simulink model "MX_Gateway"  (Simulink 9.0)
settings: Solver=FixedStepDiscrete  StartTime=0.0  StopTime=100  FixedStep=0.01  RelTol=1e-3
values from neighbouring files (symbols this model uses):
  ACTIVE = Bobcat.Parameter; CreateDefine( ACTIVE                         ,1                          ,'Define' ,'defines.h' )    [defines.m]
  ACTIVE_MODE_ONE = Bobcat.Parameter; CreateDefine( ACTIVE_MODE_ONE                ,19                         ,'Define' ,'defines.h' )    [defines.m]
  AUTHENTICATED = Bobcat.Parameter; CreateDefine( AUTHENTICATED                  ,1                          ,'Define' ,'defines.h' )    [defines.m]
  AUX_EMEA_CONTROLS = Bobcat.Parameter; CreateDefine( AUX_EMEA_CONTROLS  ,1   ,'Define' ,'defines.h' )    [enums.m]
  AUX_FLOW_HIGH = Bobcat.Parameter; CreateDefine( AUX_FLOW_HIGH           ,4  ,'Define' ,'defines.h' )    [enums.m]
  AUX_FLOW_INACTIVE = Bobcat.Parameter; CreateDefine( AUX_FLOW_INACTIVE       ,0  ,'Define' ,'defines.h' )    [enums.m]
  AUX_FLOW_LOW = Bobcat.Parameter; CreateDefine( AUX_FLOW_LOW            ,1  ,'Define' ,'defines.h' )    [enums.m]
  AUX_FLOW_MEDIUM = Bobcat.Parameter; CreateDefine( AUX_FLOW_MEDIUM         ,2  ,'Define' ,'defines.h' )    [enums.m]
  AUX_FLOW_STANDARD = Bobcat.Parameter; CreateDefine( AUX_FLOW_STANDARD       ,3  ,'Define' ,'defines.h' )    [enums.m]
  AUX_NON_FUNCTIONAL = Bobcat.Parameter; CreateDefine( AUX_NON_FUNCTIONAL ,255 ,'Define' ,'defines.h' )    [enums.m]
  BASE_ACTIVE = Bobcat.Parameter; CreateDefine( BASE_ACTIVE                    ,253                        ,'Define' ,'defines.h' )    [defines.m]
  BASE_DETENT = Bobcat.Parameter; CreateDefine( BASE_DETENT                    ,1                          ,'Define' ,'defines.h' )    [defines.m]
  BEEP_NONE = Bobcat.Parameter; CreateDefine( BEEP_NONE                      ,255                        ,'Define' ,'defines.h' )    [defines.m]
  CD_AIR_FILTER_DEBOUNCE_NORMAL = Bobcat.Parameter; CreateAliasCD( CD_AIR_FILTER_DEBOUNCE_NORMAL                        ,uint16(500)                ,'ConstantDataPointer' ,'CONSTANT_DATA' ,'AIR_FILTER_DEBOUNCE_NORMAL')    [cdata.m]    (also in hc12_cdata.m; m4_cdata.m)
  CD_AIR_FILTER_DEBOUNCE_NOT_CONNECTED = Bobcat.Parameter; CreateAliasCD( CD_AIR_FILTER_DEBOUNCE_NOT_CONNECTED                 ,uint16(3000)               ,'ConstantDataPointer' ,'CONSTANT_DATA' ,'AIR_FILTER_DEBOUNCE_NOT_CONNECTED')    [cdata.m]    (also in hc12_cdata.m; m4_cdata.m)
  CD_AIR_FILTER_DEBOUNCE_PLUGGED = Bobcat.Parameter; CreateAliasCD( CD_AIR_FILTER_DEBOUNCE_PLUGGED                       ,uint16(3000)               ,'ConstantDataPointer' ,'CONSTANT_DATA' ,'AIR_FILTER_DEBOUNCE_PLUGGED')    [cdata.m]    (also in hc12_cdata.m; m4_cdata.m)
  CD_AIR_FILTER_FUNCTION = Bobcat.Parameter; CreateAliasCD( CD_AIR_FILTER_FUNCTION                               ,uint8(255)                 ,'ConstantDataPointer' ,'CONSTANT_DATA' ,'AIR_FILTER_FUNCTION')    [cdata.m]    (also in hc12_cdata.m; m4_cdata.m)
  CD_AIR_FILTER_PLUGGED_WEIGHT = Bobcat.Parameter; CreateAliasCD( CD_AIR_FILTER_PLUGGED_WEIGHT                         ,uint8(2)                   ,'ConstantDataPointer' ,'CONSTANT_DATA' ,'AIR_FILTER_PLUGGED_WEIGHT')    [cdata.m]    (also in hc12_cdata.m; m4_cdata.m)
  CD_AIR_FILTER_UNPLUGGED_WEIGHT = Bobcat.Parameter; CreateAliasCD( CD_AIR_FILTER_UNPLUGGED_WEIGHT                       ,uint8(1)                   ,'ConstantDataPointer' ,'CONSTANT_DATA' ,'AIR_FILTER_UNPLUGGED_WEIGHT')    [cdata.m]    (also in hc12_cdata.m; m4_cdata.m)
  CD_AUTOIDLE_ARMBAR_DELAY = Bobcat.Parameter; CreateAliasCD( CD_AUTOIDLE_ARMBAR_DELAY                             ,uint8(2)                   ,'ConstantDataPointer' ,'CONSTANT_DATA' ,'AUTOIDLE_ARMBAR_DELAY')    [cdata.m]    (also in hc12_cdata.m; m4_cdata.m)
  CD_AUTOIDLE_FUNCTION = Bobcat.Parameter; CreateAliasCD( CD_AUTOIDLE_FUNCTION                                 ,uint8(255)                 ,'ConstantDataPointer' ,'CONSTANT_DATA' ,'AUTOIDLE_FUNCTION')    [cdata.m]    (also in hc12_cdata.m; m4_cdata.m)
  CD_AUTOIDLE_IDLE_DELAY = Bobcat.Parameter; CreateAliasCD( CD_AUTOIDLE_IDLE_DELAY                               ,uint8(40)                  ,'ConstantDataPointer' ,'CONSTANT_DATA' ,'AUTOIDLE_IDLE_DELAY')    [cdata.m]    (also in hc12_cdata.m; m4_cdata.m)
  CD_AUTOIDLE_LOW_IDLE_START = Bobcat.Parameter; CreateAliasCD( CD_AUTOIDLE_LOW_IDLE_START                           ,uint8(255)                 ,'ConstantDataPointer' ,'CONSTANT_DATA' ,'AUTOIDLE_LOW_IDLE_START')    [cdata.m]    (also in hc12_cdata.m; m4_cdata.m)
  CD_AUTOIDLE_PRESSURE_OFF = Bobcat.Parameter; CreateAliasCD( CD_AUTOIDLE_PRESSURE_OFF                             ,uint16(300)                ,'ConstantDataPointer' ,'CONSTANT_DATA' ,'AUTOIDLE_PRESSURE_OFF')    [cdata.m]    (also in hc12_cdata.m; m4_cdata.m)
  CD_AUTOIDLE_PRESSURE_ON = Bobcat.Parameter; CreateAliasCD( CD_AUTOIDLE_PRESSURE_ON                              ,uint16(270)                ,'ConstantDataPointer' ,'CONSTANT_DATA' ,'AUTOIDLE_PRESSURE_ON')    [cdata.m]    (also in hc12_cdata.m; m4_cdata.m)
  CD_AUTOSHIFT_FUNCTION = Bobcat.Parameter; CreateAliasCD( CD_AUTOSHIFT_FUNCTION                                ,uint8(255)                 ,'ConstantDataPointer' ,'CONSTANT_DATA' ,'AUTOSHIFT_FUNCTION')    [cdata.m]    (also in hc12_cdata.m; m4_cdata.m)
  CD_AUTOSHIFT_PHIGH1 = Bobcat.Parameter; CreateAliasCD( CD_AUTOSHIFT_PHIGH1                                  ,uint16(205)                ,'ConstantDataPointer' ,'CONSTANT_DATA' ,'AUTOSHIFT_PHIGH1')    [cdata.m]    (also in hc12_cdata.m; m4_cdata.m)
  CD_AUTOSHIFT_PHIGH2 = Bobcat.Parameter; CreateAliasCD( CD_AUTOSHIFT_PHIGH2                                  ,uint16(193)                ,'ConstantDataPointer' ,'CONSTANT_DATA' ,'AUTOSHIFT_PHIGH2')    [cdata.m]    (also in hc12_cdata.m; m4_cdata.m)
  CD_AUTOSHIFT_PHIGH_TEMP1 = Bobcat.Parameter; CreateAliasCD( CD_AUTOSHIFT_PHIGH_TEMP1                             ,int16(70)                  ,'ConstantDataPointer' ,'CONSTANT_DATA' ,'AUTOSHIFT_PHIGH_TEMP1')    [cdata.m]    (also in hc12_cdata.m; m4_cdata.m)
  CD_AUTOSHIFT_PHIGH_TEMP2 = Bobcat.Parameter; CreateAliasCD( CD_AUTOSHIFT_PHIGH_TEMP2                             ,int16(160)                 ,'ConstantDataPointer' ,'CONSTANT_DATA' ,'AUTOSHIFT_PHIGH_TEMP2')    [cdata.m]    (also in hc12_cdata.m; m4_cdata.m)
  CD_AUTOSHIFT_PLOW1 = Bobcat.Parameter; CreateAliasCD( CD_AUTOSHIFT_PLOW1                                   ,uint16(130)                ,'ConstantDataPointer' ,'CONSTANT_DATA' ,'AUTOSHIFT_PLOW1')    [cdata.m]    (also in hc12_cdata.m; m4_cdata.m)
  CD_AUTOSHIFT_PLOW2 = Bobcat.Parameter; CreateAliasCD( CD_AUTOSHIFT_PLOW2                                   ,uint16(100)                ,'ConstantDataPointer' ,'CONSTANT_DATA' ,'AUTOSHIFT_PLOW2')    [cdata.m]    (also in hc12_cdata.m; m4_cdata.m)
  CD_AUTOSHIFT_PLOW_TEMP1 = Bobcat.Parameter; CreateAliasCD( CD_AUTOSHIFT_PLOW_TEMP1                              ,int16(20)                  ,'ConstantDataPointer' ,'CONSTANT_DATA' ,'AUTOSHIFT_PLOW_TEMP1')    [cdata.m]    (also in hc12_cdata.m; m4_cdata.m)
  CD_AUTOSHIFT_PLOW_TEMP2 = Bobcat.Parameter; CreateAliasCD( CD_AUTOSHIFT_PLOW_TEMP2                              ,int16(80)                  ,'ConstantDataPointer' ,'CONSTANT_DATA' ,'AUTOSHIFT_PLOW_TEMP2')    [cdata.m]    (also in hc12_cdata.m; m4_cdata.m)
  CD_AUTOSHIFT_TRAVEL_DELAY = Bobcat.Parameter; CreateAliasCD( CD_AUTOSHIFT_TRAVEL_DELAY                            ,uint8(32)                  ,'ConstantDataPointer' ,'CONSTANT_DATA' ,'AUTOSHIFT_TRAVEL_DELAY')    [cdata.m]    (also in hc12_cdata.m; m4_cdata.m)
  CD_AUX2_CRACKPOINT_CURRENT = Bobcat.Parameter; CreateAliasCD( CD_AUX2_CRACKPOINT_CURRENT                           ,fi(0,0,16,12,'hex','0c28') ,'ConstantDataPointer' ,'CONSTANT_DATA' ,'AUX2_CRACKPOINT_CURRENT')    [cdata.m]    (also in hc12_cdata.m; m4_cdata.m)
  CD_AUX2_FLOW_LOW_FUNCTION = Bobcat.Parameter; CreateAliasCD( CD_AUX2_FLOW_LOW_FUNCTION                            ,uint8(255)                 ,'ConstantDataPointer' ,'CONSTANT_DATA' ,'AUX2_FLOW_LOW_FUNCTION')    [cdata.m]    (also in hc12_cdata.m; m4_cdata.m)
  CD_AUX2_FLOW_MED_FUNCTION = Bobcat.Parameter; CreateAliasCD( CD_AUX2_FLOW_MED_FUNCTION                            ,uint8(255)                 ,'ConstantDataPointer' ,'CONSTANT_DATA' ,'AUX2_FLOW_MED_FUNCTION')    [cdata.m]    (also in hc12_cdata.m; m4_cdata.m)
  CD_AUX2_HI_FLOW_PERCENT = Bobcat.Parameter; CreateAliasCD( CD_AUX2_HI_FLOW_PERCENT                              ,fi(0,0,16,16,'hex','ffff') ,'ConstantDataPointer' ,'CONSTANT_DATA' ,'AUX2_HI_FLOW_PERCENT')    [cdata.m]    (also in hc12_cdata.m; m4_cdata.m)
  CD_AUX2_LOW_FLOW_PERCENT = Bobcat.Parameter; CreateAliasCD( CD_AUX2_LOW_FLOW_PERCENT                             ,fi(0,0,16,16,'hex','7333') ,'ConstantDataPointer' ,'CONSTANT_DATA' ,'AUX2_LOW_FLOW_PERCENT')    [cdata.m]    (also in hc12_cdata.m; m4_cdata.m)
  CD_AUX2_MAX_FLOW_CURRENT = Bobcat.Parameter; CreateAliasCD( CD_AUX2_MAX_FLOW_CURRENT                             ,fi(0,0,16,12,'hex','14cc') ,'ConstantDataPointer' ,'CONSTANT_DATA' ,'AUX2_MAX_FLOW_CURRENT')    [cdata.m]    (also in hc12_cdata.m; m4_cdata.m)
  CD_AUX2_MED_FLOW_PERCENT = Bobcat.Parameter; CreateAliasCD( CD_AUX2_MED_FLOW_PERCENT                             ,fi(0,0,16,16,'hex','9999') ,'ConstantDataPointer' ,'CONSTANT_DATA' ,'AUX2_MED_FLOW_PERCENT')    [cdata.m]    (also in hc12_cdata.m; m4_cdata.m)
  CD_AUX_CRACKPOINT_CURRENT = Bobcat.Parameter; CreateAliasCD( CD_AUX_CRACKPOINT_CURRENT                            ,fi(0,0,16,12,'hex','0000') ,'ConstantDataPointer' ,'CONSTANT_DATA' ,'AUX_CRACKPOINT_CURRENT')    [cdata.m]    (also in hc12_cdata.m; m4_cdata.m)
  CD_AUX_DETENT_FLASH_TIME = Bobcat.Parameter; CreateAliasCD( CD_AUX_DETENT_FLASH_TIME                             ,uint16(72)                 ,'ConstantDataPointer' ,'CONSTANT_DATA' ,'AUX_DETENT_FLASH_TIME')    [cdata.m]    (also in hc12_cdata.m; m4_cdata.m)
  CD_AUX_DETENT_HOLD_TIME = Bobcat.Parameter; CreateAliasCD( CD_AUX_DETENT_HOLD_TIME                              ,uint16(100)                ,'ConstantDataPointer' ,'CONSTANT_DATA' ,'AUX_DETENT_HOLD_TIME')    [cdata.m]    (also in hc12_cdata.m; m4_cdata.m)
  CD_AUX_FULL_ON_AUX1 = Bobcat.Parameter; CreateAliasCD( CD_AUX_FULL_ON_AUX1                                  ,fi(0,0,16,12,'hex','10cd') ,'ConstantDataPointer' ,'CONSTANT_DATA' ,'AUX_FULL_ON_AUX1')    [cdata.m]    (also in hc12_cdata.m; m4_cdata.m)
  CD_AUX_FULL_ON_AUX2 = Bobcat.Parameter; CreateAliasCD( CD_AUX_FULL_ON_AUX2                                  ,fi(0,0,16,12,'hex','139d') ,'ConstantDataPointer' ,'CONSTANT_DATA' ,'AUX_FULL_ON_AUX2')    [cdata.m]    (also in hc12_cdata.m; m4_cdata.m)
  CD_AUX_FULL_ON_AUX3 = Bobcat.Parameter; CreateAliasCD( CD_AUX_FULL_ON_AUX3                                  ,fi(0,0,16,12,'hex','17d7') ,'ConstantDataPointer' ,'CONSTANT_DATA' ,'AUX_FULL_ON_AUX3')    [cdata.m]    (also in hc12_cdata.m; m4_cdata.m)
  CD_AUX_FUNCTION = Bobcat.Parameter; CreateAliasCD( CD_AUX_FUNCTION                                      ,uint8(0)                   ,'ConstantDataPointer' ,'CONSTANT_DATA' ,'AUX_FUNCTION')    [cdata.m]    (also in hc12_cdata.m; m4_cdata.m)
  CD_AUX_RATE_LIMIT_CRACKPOINT = Bobcat.Parameter; CreateAliasCD( CD_AUX_RATE_LIMIT_CRACKPOINT                         ,fi(0,0,16,12,'hex','0800') ,'ConstantDataPointer' ,'CONSTANT_DATA' ,'AUX_RATE_LIMIT_CRACKPOINT')    [cdata.m]    (also in hc12_cdata.m; m4_cdata.m)
  CD_AUX_RATE_LIMIT_CRACKPOINT_RATE = Bobcat.Parameter; CreateAliasCD( CD_AUX_RATE_LIMIT_CRACKPOINT_RATE                    ,fi(0,1,32,12,'int',102400) ,'ConstantDataPointer' ,'CONSTANT_DATA' ,'AUX_RATE_LIMIT_CRACKPOINT_RATE')    [cdata.m]    (also in hc12_cdata.m; m4_cdata.m)
  CD_AUX_RATE_LIMIT_FUNCTION = Bobcat.Parameter; CreateAliasCD( CD_AUX_RATE_LIMIT_FUNCTION                           ,uint8(255)                 ,'ConstantDataPointer' ,'CONSTANT_DATA' ,'AUX_RATE_LIMIT_FUNCTION')    [cdata.m]    (also in hc12_cdata.m; m4_cdata.m)
  CD_AUX_RATE_LIMIT_MAX_ENG_SPD = Bobcat.Parameter; CreateAliasCD( CD_AUX_RATE_LIMIT_MAX_ENG_SPD                        ,uint16(1600)               ,'ConstantDataPointer' ,'CONSTANT_DATA' ,'AUX_RATE_LIMIT_MAX_ENG_SPD')    [cdata.m]    (also in hc12_cdata.m; m4_cdata.m)
  CD_AUX_RATE_LIMIT_MIN_ENG_SPD = Bobcat.Parameter; CreateAliasCD( CD_AUX_RATE_LIMIT_MIN_ENG_SPD                        ,uint16(100)                ,'ConstantDataPointer' ,'CONSTANT_DATA' ,'AUX_RATE_LIMIT_MIN_ENG_SPD')    [cdata.m]    (also in hc12_cdata.m; m4_cdata.m)
  CD_AUX_RATE_LIMIT_NORMAL_RATE = Bobcat.Parameter; CreateAliasCD( CD_AUX_RATE_LIMIT_NORMAL_RATE                        ,fi(0,1,32,12,'int',2048)   ,'ConstantDataPointer' ,'CONSTANT_DATA' ,'AUX_RATE_LIMIT_NORMAL_RATE')    [cdata.m]    (also in hc12_cdata.m; m4_cdata.m)
  CD_AUXPOT_CAL_DEADBAND_HIGH = Bobcat.Parameter; CreateAliasCD( CD_AUXPOT_CAL_DEADBAND_HIGH                          ,uint16(574)                ,'ConstantDataPointer' ,'CONSTANT_DATA' ,'AUXPOT_CAL_DEADBAND_HIGH')    [cdata.m]    (also in hc12_cdata.m; m4_cdata.m)
  CD_AUXPOT_CAL_DEADBAND_LOW = Bobcat.Parameter; CreateAliasCD( CD_AUXPOT_CAL_DEADBAND_LOW                           ,uint16(424)                ,'ConstantDataPointer' ,'CONSTANT_DATA' ,'AUXPOT_CAL_DEADBAND_LOW')    [cdata.m]    (also in hc12_cdata.m; m4_cdata.m)
  CD_AUXPOT_CENTER_RATIO = Bobcat.Parameter; CreateAliasCD( CD_AUXPOT_CENTER_RATIO                               ,uint16(500)                ,'ConstantDataPointer' ,'CONSTANT_DATA' ,'AUXPOT_CENTER_RATIO')    [cdata.m]    (also in hc12_cdata.m; m4_cdata.m)
  CD_AUXPOT_DEADBAND = Bobcat.Parameter; CreateAliasCD( CD_AUXPOT_DEADBAND                                   ,uint8(38)                  ,'ConstantDataPointer' ,'CONSTANT_DATA' ,'AUXPOT_DEADBAND')    [cdata.m]    (also in hc12_cdata.m; m4_cdata.m)
  CD_AUXPOT_FUNCTION = Bobcat.Parameter; CreateAliasCD( CD_AUXPOT_FUNCTION                                   ,uint8(0)                   ,'ConstantDataPointer' ,'CONSTANT_DATA' ,'AUXPOT_FUNCTION')    [cdata.m]    (also in hc12_cdata.m; m4_cdata.m)
  CD_AUXPOT_RATIO_GAIN = Bobcat.Parameter; CreateAliasCD( CD_AUXPOT_RATIO_GAIN                                 ,uint16(1000)               ,'ConstantDataPointer' ,'CONSTANT_DATA' ,'AUXPOT_RATIO_GAIN')    [cdata.m]    (also in hc12_cdata.m; m4_cdata.m)
  CD_CAN_THROTTLE_FUNCTION = Bobcat.Parameter; CreateAliasCD( CD_CAN_THROTTLE_FUNCTION                             ,uint8(255)                 ,'ConstantDataPointer' ,'CONSTANT_DATA' ,'CAN_THROTTLE_FUNCTION')    [cdata.m]    (also in hc12_cdata.m; m4_cdata.m)
  CD_CAN_THROTTLE_REVERSE = Bobcat.Parameter; CreateAliasCD( CD_CAN_THROTTLE_REVERSE                              ,uint8(255)                 ,'ConstantDataPointer' ,'CONSTANT_DATA' ,'CAN_THROTTLE_REVERSE')    [cdata.m]    (also in hc12_cdata.m; m4_cdata.m)
  CD_CONSOLE_DEBOUNCE_ABSENT = Bobcat.Parameter; CreateAliasCD( CD_CONSOLE_DEBOUNCE_ABSENT                           ,uint16(12)                 ,'ConstantDataPointer' ,'CONSTANT_DATA' ,'CONSOLE_DEBOUNCE_ABSENT')    [cdata.m]    (also in hc12_cdata.m; m4_cdata.m)
  CD_CONSOLE_DEBOUNCE_OORH = Bobcat.Parameter; CreateAliasCD( CD_CONSOLE_DEBOUNCE_OORH                             ,uint16(12)                 ,'ConstantDataPointer' ,'CONSTANT_DATA' ,'CONSOLE_DEBOUNCE_OORH')    [cdata.m]    (also in hc12_cdata.m; m4_cdata.m)
  CD_CONSOLE_DEBOUNCE_OORL = Bobcat.Parameter; CreateAliasCD( CD_CONSOLE_DEBOUNCE_OORL                             ,uint16(12)                 ,'ConstantDataPointer' ,'CONSTANT_DATA' ,'CONSOLE_DEBOUNCE_OORL')    [cdata.m]    (also in hc12_cdata.m; m4_cdata.m)
  CD_CONSOLE_DEBOUNCE_PRESENT = Bobcat.Parameter; CreateAliasCD( CD_CONSOLE_DEBOUNCE_PRESENT                          ,uint16(12)                 ,'ConstantDataPointer' ,'CONSTANT_DATA' ,'CONSOLE_DEBOUNCE_PRESENT')    [cdata.m]    (also in hc12_cdata.m; m4_cdata.m)
  CD_CONSOLE_FUNCTION = Bobcat.Parameter; CreateAliasCD( CD_CONSOLE_FUNCTION                                  ,uint8(0)                   ,'ConstantDataPointer' ,'CONSTANT_DATA' ,'CONSOLE_FUNCTION')    [cdata.m]    (also in hc12_cdata.m; m4_cdata.m)
  CD_CONSOLE_OORH = Bobcat.Parameter; CreateAliasCD( CD_CONSOLE_OORH                                      ,uint16(740)                ,'ConstantDataPointer' ,'CONSTANT_DATA' ,'CONSOLE_OORH')    [cdata.m]    (also in hc12_cdata.m; m4_cdata.m)
  CD_CONSOLE_OORL = Bobcat.Parameter; CreateAliasCD( CD_CONSOLE_OORL                                      ,uint16(10)                 ,'ConstantDataPointer' ,'CONSTANT_DATA' ,'CONSOLE_OORL')    [cdata.m]    (also in hc12_cdata.m; m4_cdata.m)
  CD_CONSOLE_PRESENT_HIGH = Bobcat.Parameter; CreateAliasCD( CD_CONSOLE_PRESENT_HIGH                              ,uint16(515)                ,'ConstantDataPointer' ,'CONSTANT_DATA' ,'CONSOLE_PRESENT_HIGH')    [cdata.m]    (also in hc12_cdata.m; m4_cdata.m)
  CD_CONSOLE_PRESENT_LOW = Bobcat.Parameter; CreateAliasCD( CD_CONSOLE_PRESENT_LOW                               ,uint16(375)                ,'ConstantDataPointer' ,'CONSTANT_DATA' ,'CONSOLE_PRESENT_LOW')    [cdata.m]    (also in hc12_cdata.m; m4_cdata.m)
  CD_DELUXE_GEN5_5_FUNCTION = Bobcat.Parameter; CreateAliasCD( CD_DELUXE_GEN5_5_FUNCTION                            ,uint8(255)                 ,'ConstantDataPointer' ,'CONSTANT_DATA' ,'DELUXE_GEN5_5_FUNCTION')    [cdata.m]    (also in hc12_cdata.m; m4_cdata.m)
  CD_DELUXE_GEN5_FUNCTION = Bobcat.Parameter; CreateAliasCD( CD_DELUXE_GEN5_FUNCTION                              ,uint8(255)                 ,'ConstantDataPointer' ,'CONSTANT_DATA' ,'DELUXE_GEN5_FUNCTION')    [cdata.m]    (also in hc12_cdata.m; m4_cdata.m)
  CD_DERATE_ENGINE_SPEED_DEBOUNCE_HIGH = Bobcat.Parameter; CreateAliasCD( CD_DERATE_ENGINE_SPEED_DEBOUNCE_HIGH                 ,uint16(200)                ,'ConstantDataPointer' ,'CONSTANT_DATA' ,'DERATE_ENGINE_SPEED_DEBOUNCE_HIGH')    [cdata.m]    (also in hc12_cdata.m; m4_cdata.m)
  CD_DERATE_ENGINE_SPEED_DEBOUNCE_SHUTDOWN = Bobcat.Parameter; CreateAliasCD( CD_DERATE_ENGINE_SPEED_DEBOUNCE_SHUTDOWN             ,uint16(1000)               ,'ConstantDataPointer' ,'CONSTANT_DATA' ,'DERATE_ENGINE_SPEED_DEBOUNCE_SHUTDOWN')    [cdata.m]    (also in hc12_cdata.m; m4_cdata.m)
  CD_DERATE_ENGINE_SPEED_HIGH_OFFSET = Bobcat.Parameter; CreateAliasCD( CD_DERATE_ENGINE_SPEED_HIGH_OFFSET                   ,uint16(200)                ,'ConstantDataPointer' ,'CONSTANT_DATA' ,'DERATE_ENGINE_SPEED_HIGH_OFFSET')    [cdata.m]    (also in hc12_cdata.m; m4_cdata.m)
  CD_DERATE_METHOD = Bobcat.Parameter; CreateAliasCD( CD_DERATE_METHOD                                     ,uint8(0)                   ,'ConstantDataPointer' ,'CONSTANT_DATA' ,'DERATE_METHOD')    [cdata.m]    (also in hc12_cdata.m; m4_cdata.m)
  CD_DUMP_VALVE_FUNCTION = Bobcat.Parameter; CreateAliasCD( CD_DUMP_VALVE_FUNCTION                               ,uint8(255)                 ,'ConstantDataPointer' ,'CONSTANT_DATA' ,'DUMP_VALVE_FUNCTION')    [cdata.m]    (also in hc12_cdata.m; m4_cdata.m)
  CD_DUMP_VALVE_ON_CURRENT = Bobcat.Parameter; CreateAliasCD( CD_DUMP_VALVE_ON_CURRENT                             ,fi(0,0,16,12,'hex','ffff') ,'ConstantDataPointer' ,'CONSTANT_DATA' ,'DUMP_VALVE_ON_CURRENT')    [cdata.m]    (also in hc12_cdata.m; m4_cdata.m)
  CD_ECO_MODE_THROTTLE_REDUCTION = Bobcat.Parameter; CreateAliasCD( CD_ECO_MODE_THROTTLE_REDUCTION                       ,uint16(850)                ,'ConstantDataPointer' ,'CONSTANT_DATA' ,'ECO_MODE_THROTTLE_REDUCTION')    [cdata.m]    (also in hc12_cdata.m; m4_cdata.m)
  CD_ECT_FILTER_GAIN = Bobcat.Parameter; CreateAliasCD( CD_ECT_FILTER_GAIN                                   ,uint8(6)                   ,'ConstantDataPointer' ,'CONSTANT_DATA' ,'ECT_FILTER_GAIN')    [cdata.m]    (also in hc12_cdata.m; m4_cdata.m)
  CD_ECU_FUNCTION = Bobcat.Parameter; CreateAliasCD( CD_ECU_FUNCTION                                      ,uint8(255)                 ,'ConstantDataPointer' ,'CONSTANT_DATA' ,'ECU_FUNCTION')    [cdata.m]    (also in hc12_cdata.m; m4_cdata.m)
  CD_ECU_HOLD_DEBOUNCE_ERROR_ON = Bobcat.Parameter; CreateAliasCD( CD_ECU_HOLD_DEBOUNCE_ERROR_ON                        ,uint16(20)                 ,'ConstantDataPointer' ,'CONSTANT_DATA' ,'ECU_HOLD_DEBOUNCE_ERROR_ON')    [cdata.m]    (also in hc12_cdata.m; m4_cdata.m)
  CD_EMEA_CONTROLS_HOLD_TIME = Bobcat.Parameter; CreateAliasCD( CD_EMEA_CONTROLS_HOLD_TIME                           ,uint16(30)                 ,'ConstantDataPointer' ,'CONSTANT_DATA' ,'EMEA_CONTROLS_HOLD_TIME')    [cdata.m]    (also in hc12_cdata.m; m4_cdata.m)
  CD_EMEA_CONTROLS_OPTION_A = Bobcat.Parameter; CreateAliasCD( CD_EMEA_CONTROLS_OPTION_A                            ,uint8(255)                 ,'ConstantDataPointer' ,'CONSTANT_DATA' ,'EMEA_CONTROLS_OPTION_A')    [cdata.m]    (also in hc12_cdata.m; m4_cdata.m)
  CD_EMEA_CONTROLS_OPTION_C = Bobcat.Parameter; CreateAliasCD( CD_EMEA_CONTROLS_OPTION_C                            ,uint8(255)                 ,'ConstantDataPointer' ,'CONSTANT_DATA' ,'EMEA_CONTROLS_OPTION_C')    [cdata.m]    (also in hc12_cdata.m; m4_cdata.m)
  CD_EMEA_CONTROLS_REVERSE = Bobcat.Parameter; CreateAliasCD( CD_EMEA_CONTROLS_REVERSE                             ,uint8(255)                 ,'ConstantDataPointer' ,'CONSTANT_DATA' ,'EMEA_CONTROLS_REVERSE')    [cdata.m]    (also in hc12_cdata.m; m4_cdata.m)
  CD_EMEA_ELECTRIC_VERSION = Bobcat.Parameter; CreateAliasCD( CD_EMEA_ELECTRIC_VERSION                             ,uint8(0)                   ,'ConstantDataPointer' ,'CONSTANT_DATA' ,'EMEA_ELECTRIC_VERSION')    [cdata.m]    (also in hc12_cdata.m; m4_cdata.m)
  CD_EMEA_POT_CAL_DEADBAND_HIGH = Bobcat.Parameter; CreateAliasCD( CD_EMEA_POT_CAL_DEADBAND_HIGH                        ,uint16(574)                ,'ConstantDataPointer' ,'CONSTANT_DATA' ,'EMEA_POT_CAL_DEADBAND_HIGH')    [cdata.m]    (also in hc12_cdata.m; m4_cdata.m)
  CD_EMEA_POT_CAL_DEADBAND_LOW = Bobcat.Parameter; CreateAliasCD( CD_EMEA_POT_CAL_DEADBAND_LOW                         ,uint16(424)                ,'ConstantDataPointer' ,'CONSTANT_DATA' ,'EMEA_POT_CAL_DEADBAND_LOW')    [cdata.m]    (also in hc12_cdata.m; m4_cdata.m)
  CD_ENGINE_RPM_FACTOR = Bobcat.Parameter; CreateAliasCD( CD_ENGINE_RPM_FACTOR                                 ,uint8(35)                  ,'ConstantDataPointer' ,'CONSTANT_DATA' ,'ENGINE_RPM_FACTOR')    [cdata.m]    (also in hc12_cdata.m; m4_cdata.m)
  CD_ENGINE_SPEED_FILTER_FAST_VALUE = Bobcat.Parameter; CreateAliasCD( CD_ENGINE_SPEED_FILTER_FAST_VALUE                    ,uint8(1)                   ,'ConstantDataPointer' ,'CONSTANT_DATA' ,'ENGINE_SPEED_FILTER_FAST_VALUE')    [cdata.m]    (also in hc12_cdata.m; m4_cdata.m)
  CD_ENGINE_SPEED_FILTER_SLOW_VALUE = Bobcat.Parameter; CreateAliasCD( CD_ENGINE_SPEED_FILTER_SLOW_VALUE                    ,uint8(4)                   ,'ConstantDataPointer' ,'CONSTANT_DATA' ,'ENGINE_SPEED_FILTER_SLOW_VALUE')    [cdata.m]    (also in hc12_cdata.m; m4_cdata.m)
  CD_ENGINE_SPEED_RUNNING = Bobcat.Parameter; CreateAliasCD( CD_ENGINE_SPEED_RUNNING                              ,uint16(800)                ,'ConstantDataPointer' ,'CONSTANT_DATA' ,'ENGINE_SPEED_RUNNING')    [cdata.m]    (also in hc12_cdata.m; m4_cdata.m)
  CD_ENGINE_START_DELAY_DEBOUNCE_RPM_NO_SIGNAL = Bobcat.Parameter; CreateAliasCD( CD_ENGINE_START_DELAY_DEBOUNCE_RPM_NO_SIGNAL         ,uint16(0)                  ,'ConstantDataPointer' ,'CONSTANT_DATA' ,'ENGINE_START_DELAY_DEBOUNCE_RPM_NO_SIGNAL')    [cdata.m]    (also in hc12_cdata.m; m4_cdata.m)
  CD_ENGINE_START_DELAY_OFF_TIME = Bobcat.Parameter; CreateAliasCD( CD_ENGINE_START_DELAY_OFF_TIME                       ,uint16(0)                  ,'ConstantDataPointer' ,'CONSTANT_DATA' ,'ENGINE_START_DELAY_OFF_TIME')    [cdata.m]    (also in hc12_cdata.m; m4_cdata.m)
  CD_ENGINE_START_DELAY_RPM_NO_SIGNAL = Bobcat.Parameter; CreateAliasCD( CD_ENGINE_START_DELAY_RPM_NO_SIGNAL                  ,uint16(0)                  ,'ConstantDataPointer' ,'CONSTANT_DATA' ,'ENGINE_START_DELAY_RPM_NO_SIGNAL')    [cdata.m]    (also in hc12_cdata.m; m4_cdata.m)
  CD_EOP_DEBOUNCE_EXTREMELY_LOW = Bobcat.Parameter; CreateAliasCD( CD_EOP_DEBOUNCE_EXTREMELY_LOW                        ,uint16(50)                 ,'ConstantDataPointer' ,'CONSTANT_DATA' ,'EOP_DEBOUNCE_EXTREMELY_LOW')    [cdata.m]    (also in hc12_cdata.m; m4_cdata.m)
  CD_EOP_DEBOUNCE_NORMAL = Bobcat.Parameter; CreateAliasCD( CD_EOP_DEBOUNCE_NORMAL                               ,uint16(50)                 ,'ConstantDataPointer' ,'CONSTANT_DATA' ,'EOP_DEBOUNCE_NORMAL')    [cdata.m]    (also in hc12_cdata.m; m4_cdata.m)
  CD_EOP_DEBOUNCE_SHUTDOWN = Bobcat.Parameter; CreateAliasCD( CD_EOP_DEBOUNCE_SHUTDOWN                             ,uint16(100)                ,'ConstantDataPointer' ,'CONSTANT_DATA' ,'EOP_DEBOUNCE_SHUTDOWN')    [cdata.m]    (also in hc12_cdata.m; m4_cdata.m)
  CD_EOP_FILTER_VALUE = Bobcat.Parameter; CreateAliasCD( CD_EOP_FILTER_VALUE                                  ,uint8(3)                   ,'ConstantDataPointer' ,'CONSTANT_DATA' ,'EOP_FILTER_VALUE')    [cdata.m]    (also in hc12_cdata.m; m4_cdata.m)
  CD_EOP_FUNCTION = Bobcat.Parameter; CreateAliasCD( CD_EOP_FUNCTION                                      ,uint8(1)                   ,'ConstantDataPointer' ,'CONSTANT_DATA' ,'EOP_FUNCTION')    [cdata.m]    (also in hc12_cdata.m; m4_cdata.m)
  CD_EOP_SWITCH_GAUGE_VALUE = Bobcat.Parameter; CreateAliasCD( CD_EOP_SWITCH_GAUGE_VALUE                            ,uint16(125)                ,'ConstantDataPointer' ,'CONSTANT_DATA' ,'EOP_SWITCH_GAUGE_VALUE')    [cdata.m]    (also in hc12_cdata.m; m4_cdata.m)
  CD_EOP_SWITCH_LOW = Bobcat.Parameter; CreateAliasCD( CD_EOP_SWITCH_LOW                                    ,uint16(50)                 ,'ConstantDataPointer' ,'CONSTANT_DATA' ,'EOP_SWITCH_LOW')    [cdata.m]    (also in hc12_cdata.m; m4_cdata.m)
  CD_EOP_SWITCH_PRESSURE_VALUE = Bobcat.Parameter; CreateAliasCD( CD_EOP_SWITCH_PRESSURE_VALUE                         ,uint16(40)                 ,'ConstantDataPointer' ,'CONSTANT_DATA' ,'EOP_SWITCH_PRESSURE_VALUE')    [cdata.m]    (also in hc12_cdata.m; m4_cdata.m)
  CD_EOT_DEBOUNCE_EXTREMELY_LOW = Bobcat.Parameter; CreateAliasCD( CD_EOT_DEBOUNCE_EXTREMELY_LOW                        ,uint16(2)                  ,'ConstantDataPointer' ,'CONSTANT_DATA' ,'EOT_DEBOUNCE_EXTREMELY_LOW')    [cdata.m]    (also in hc12_cdata.m; m4_cdata.m)
  CD_EOT_DEBOUNCE_LOW = Bobcat.Parameter; CreateAliasCD( CD_EOT_DEBOUNCE_LOW                                  ,uint16(2)                  ,'ConstantDataPointer' ,'CONSTANT_DATA' ,'EOT_DEBOUNCE_LOW')    [cdata.m]    (also in hc12_cdata.m; m4_cdata.m)
  CD_EOT_DEBOUNCE_NORMAL = Bobcat.Parameter; CreateAliasCD( CD_EOT_DEBOUNCE_NORMAL                               ,uint16(2)                  ,'ConstantDataPointer' ,'CONSTANT_DATA' ,'EOT_DEBOUNCE_NORMAL')    [cdata.m]    (also in hc12_cdata.m; m4_cdata.m)
  CD_EOT_EXTREMELY_LOW = Bobcat.Parameter; CreateAliasCD( CD_EOT_EXTREMELY_LOW                                 ,uint16(81)                 ,'ConstantDataPointer' ,'CONSTANT_DATA' ,'EOT_EXTREMELY_LOW')    [cdata.m]    (also in hc12_cdata.m; m4_cdata.m)
  CD_EOT_FUNCTION = Bobcat.Parameter; CreateAliasCD( CD_EOT_FUNCTION                                      ,uint8(255)                 ,'ConstantDataPointer' ,'CONSTANT_DATA' ,'EOT_FUNCTION')    [cdata.m]    (also in hc12_cdata.m; m4_cdata.m)
  CD_EOT_LOW = Bobcat.Parameter; CreateAliasCD( CD_EOT_LOW                                           ,uint16(90)                 ,'ConstantDataPointer' ,'CONSTANT_DATA' ,'EOT_LOW')    [cdata.m]    (also in hc12_cdata.m; m4_cdata.m)
  CD_EOT_TEMPERATURE_HYSTERESIS = Bobcat.Parameter; CreateAliasCD( CD_EOT_TEMPERATURE_HYSTERESIS                        ,uint16(2)                  ,'ConstantDataPointer' ,'CONSTANT_DATA' ,'EOT_TEMPERATURE_HYSTERESIS')    [cdata.m]    (also in hc12_cdata.m; m4_cdata.m)
  CD_FAST_TRAVEL_FUNCTION = Bobcat.Parameter; CreateAliasCD( CD_FAST_TRAVEL_FUNCTION                              ,uint8(255)                 ,'ConstantDataPointer' ,'CONSTANT_DATA' ,'FAST_TRAVEL_FUNCTION')    [cdata.m]    (also in hc12_cdata.m; m4_cdata.m)
  CD_FAST_TRAVEL_THROTTLE_DELAY = Bobcat.Parameter; CreateAliasCD( CD_FAST_TRAVEL_THROTTLE_DELAY                        ,uint16(10)                 ,'ConstantDataPointer' ,'CONSTANT_DATA' ,'FAST_TRAVEL_THROTTLE_DELAY')    [cdata.m]    (also in hc12_cdata.m; m4_cdata.m)
  CD_FAST_TRAVEL_THROTTLE_LIMIT = Bobcat.Parameter; CreateAliasCD( CD_FAST_TRAVEL_THROTTLE_LIMIT                        ,uint16(1000)               ,'ConstantDataPointer' ,'CONSTANT_DATA' ,'FAST_TRAVEL_THROTTLE_LIMIT')    [cdata.m]    (also in hc12_cdata.m; m4_cdata.m)
  CD_FLYWHEEL_TEETH = Bobcat.Parameter; CreateAliasCD( CD_FLYWHEEL_TEETH                                    ,uint8(83)                  ,'ConstantDataPointer' ,'CONSTANT_DATA' ,'FLYWHEEL_TEETH')    [cdata.m]    (also in hc12_cdata.m; m4_cdata.m)
  CD_FUEL_LEVEL_FILTER_FAST_VALUE = Bobcat.Parameter; CreateAliasCD( CD_FUEL_LEVEL_FILTER_FAST_VALUE                      ,uint8(5)                   ,'ConstantDataPointer' ,'CONSTANT_DATA' ,'FUEL_LEVEL_FILTER_FAST_VALUE')    [cdata.m]    (also in hc12_cdata.m; m4_cdata.m)
  CD_FUEL_LEVEL_FILTER_SLOW_VALUE = Bobcat.Parameter; CreateAliasCD( CD_FUEL_LEVEL_FILTER_SLOW_VALUE                      ,uint8(10)                  ,'ConstantDataPointer' ,'CONSTANT_DATA' ,'FUEL_LEVEL_FILTER_SLOW_VALUE')    [cdata.m]    (also in hc12_cdata.m; m4_cdata.m)
  CD_FUEL_PRIMARY_FEEDBACK_FUNCTION = Bobcat.Parameter; CreateAliasCD( CD_FUEL_PRIMARY_FEEDBACK_FUNCTION                    ,uint8(0)                   ,'ConstantDataPointer' ,'CONSTANT_DATA' ,'FUEL_PRIMARY_FEEDBACK_FUNCTION')    [cdata.m]    (also in hc12_cdata.m; m4_cdata.m)
  ... (+246 more)
undefined symbols (not defined in the file or in its folder): sigbuilder_block
undefined symbols (not defined in the file): CD_FUEL_RESOLUTION_TIMER
libraries: simulink x186, MX_Library x107
(part 3 of 48)

== system MX_Gateway/MX_Gtwy_Control ==
  bus_Input : Inport
  Error_Codes_Array : Subsystem
  Inputs : Subsystem
  Outputs : Subsystem
  PanelInterface : Subsystem
  System : Subsystem
  bus_Internal_Input : Outport
  bus_System : Outport  Port=2
  bus_Output : Outport  Port=3
  bus_Panel : Outport  Port=4
  Error_Codes : Outport  Port=5
  wires:
  [bus_Internal_Input] -> bus_Internal_Input/1  "bus_Internal_Input"
  [bus_System] -> bus_System/1  "bus_System"
  [bus_Output] -> bus_Output/1  "bus_Output"
  [bus_Panel] -> bus_Panel/1  "bus_Panel"
  [bus_Internal_Input] -> PanelInterface/2(bus_Internal_Input)  "bus_Internal_Inputs"
  [bus_System] -> PanelInterface/1(bus_System)  "bus_System"
  PanelInterface/1(bus_Panel) -> [bus_Panel]  "bus_Panel"
  bus_Input/1 -> [bus_Input]  "bus_Input"
  [bus_Input] -> Inputs/1(bus_Input)  "bus_Input"
  [bus_System] -> Inputs/2(bus_System)  "bus_System"
  Inputs/1(bus_Internal_Input) -> [bus_Internal_Input]  "bus_Internal_Input"
  [bus_Internal_Input] -> System/1(bus_Internal_Input)  "bus_Internal_Inputs"
  System/1(bus_System) -> [bus_System]  "bus_System"
  [bus_Output] -> System/2(bus_Output)  "bus_Output"
  [bus_Input] -> System/3(bus_Input)  "bus_Input"
  Outputs/1(bus_Output) -> [bus_Output]  "bus_Output"
  [bus_Input] -> Outputs/1(bus_Input)  "bus_Input"
  [bus_System] -> Outputs/2(bus_System)  "bus_System"
  [bus_Internal_Input] -> Outputs/3(bus_Internal_Input)  "bus_Internal_Inputs"
  [Error_Codes] -> Error_Codes/1  "Error_Codes"
  [bus_Internal_Input] -> Error_Codes_Array/1(bus_Internal_Input)  "bus_Internal_Input"
  [bus_Input] -> Error_Codes_Array/2(bus_Input)  "bus_Input"
  [bus_Output] -> Error_Codes_Array/3(bus_Output)  "bus_Output"
  Error_Codes_Array/1(ECodes) -> [Error_Codes]  "Error_Codes"
  [bus_Output] -> PanelInterface/3(bus_Output)  "bus_Output"
  [bus_Input] -> PanelInterface/4(bus_Input)  "bus_Input"
  [bus_System] -> Error_Codes_Array/4(bus_System)  "bus_System"
  [bus_Output] -> Inputs/3(bus_Output)
